simulink model "ofdm_txrx_alamouti"  (Simulink 6.3)
settings: Solver=ode45  StartTime=0.0  StopTime=45000  RelTol=1e-3
InitFcn:
  | ofdm_rx_alamouti_init
StartFcn:
  | ofdm_rx_alamouti_init
values from neighbouring files (symbols this model uses):
  CPLength = 16    [ofdm_tx_alamouti_init.m]
  CRCPolynomial = hex2dec('04c11db7')    [calcTxCRC.m]    (also in ofdm_tx_alamouti_init.m)
  hi = zeros(1,length(cci))    [ofdm_rx_alamouti_init.m]
  hr = zeros(1,length(ccr))    [ofdm_rx_alamouti_init.m]
  max_CP_length = 16    [ofdm_tx_alamouti_init.m]
  max_num_baseRateSymbols = 31    [ofdm_tx_alamouti_init.m]
  max_num_subcarriers = 64    [ofdm_tx_alamouti_init.m]
  max_num_trainingSymbols = 15    [ofdm_tx_alamouti_init.m]
  max_OFDM_symbols = 2047    [ofdm_tx_alamouti_init.m]
  modConstellation_qam16 = 0.75*[1 3 -1 -3]./3    [ofdm_tx_alamouti_init.m]
  modConstellation_qam256 = 0.9375*[3 1 5 7 11 9 13 15 -3 -1 -5 -7 -11 -9 -13 -15]./15    [ofdm_tx_alamouti_init.m]
  modConstellation_qam64 = 0.875*[3 1 5 7 -3 -1 -5 -7]./7    [ofdm_tx_alamouti_init.m]
  modConstellation_qpsk = [1 -1]./sqrt(2)    [ofdm_tx_alamouti_init.m]
  numBaseRateSymbols = 2    [ofdm_tx_alamouti_init.m]
  numFullRateSymbols = numFullRateSymbols + mod(numFullRateSymbols, 2)    [ofdm_tx_alamouti_init.m]
  numSubcarriers = 64    [ofdm_tx_alamouti_init.m]
  numTrainingSymbols = 4    [ofdm_tx_alamouti_init.m]
  pkt_crossCorr_thresh = 0.7    [ofdm_rx_alamouti_init.m]
  pkt_energy_thresh = 0    [ofdm_rx_alamouti_init.m]
  pkt_numPayloadBytes = 24+4+20+20+500    (= 568)    [ofdm_tx_alamouti_init.m]
  pktTiming_count_payloadStart = pktTiming_count_lsStart + pktTiming_ls_num*pktTiming_ls_length    [ofdm_rx_init_packetTimingControl.m]
  preamble = 6*[0 shortsyms_10 longsyms_2]    [ofdm_tx_alamouti_init.m]
  preamble_I = real(preamble)    [ofdm_tx_alamouti_init.m]
  preamble_Q = imag(preamble)    [ofdm_tx_alamouti_init.m]
  RAM_init_size = 4096    [ofdm_tx_alamouti_init.m]
  rx_longCorrCountLoad = 251-16    (= 235)    [ofdm_rx_alamouti_init.m]
  rx_longCorrThresh = 3000    [ofdm_rx_alamouti_init.m]
  RxDataScrambling_Seq = [40 198 78 63 82 173 102 245 48 111 172 115 147 230 216 93 72 65 62 2 205 242 122 90 128 83 105 97 73 10 5 252]    [ofdm_tx_alamouti_init.m]
  simOnly_numSamples = length(preamble)+( (numSubcarriers+CPLength)*(numTrainingSymbols + numBaseRateSymbols + numFullRateSymbols) )    [ofdm_tx_alamouti_init.m]
  train = [train train]    [ofdm_tx_alamouti_init.m]
  TxDataScrambling_Seq = [40 198 78 63 82 173 102 245 48 111 172 115 147 230 216 93 72 65 62 2 205 242 122 90 128 83 105 97 73 10 5 252]    [ofdm_tx_alamouti_init.m]
undefined symbols (not defined in the file or in its folder): CRC_table_gen, gcbh, interpFilter_4, xlBlockLoadCallback
libraries: xbsIndex_r4 x4373, dspmlti4 x4, dspsigops x4, commchan3 x2, simulink x2, commrflib2 x2, commsink2 x2, dspsrcs4 x1
(part 6 of 39)

== system ofdm_txrx_alamouti/OFDM Rx Alamouti/Equalizer & Packetizer/Packet_Constructor ==
  note: The index keeps track of the first unused location in the buffer, indexed from zero.
      |   I.e. if there are 5 bits in the buffer occupying 0 to 4, the index will be 5.
      | This is used to select which byte out of the 15 bits in the buffer will make it into the RAM block.
  I : Inport
  Q : Inport  Port=2
  Xk_index : Inport  Port=3
  Vin : Inport  Port=4
  Sym Index : Inport  Port=5
  Start : Inport  Port=6
  "  " : Subsystem  mask{period=1}
  >=8? : xbsIndex_r4/Slice  Orientation=up  nbits=1  boolean_output=off  mode=Lower Bit Location + Width  bit1=0  base1=MSB of Input  bit0=3  base0=LSB of Input  dbl_ovrd=off  block_type=slice
      settings: infoedit="Extracts a given range of bits from each input sample and presents it at the output.  The output type is ordinarily unsigned with binary point at zero, but can be Boolean when the slice is one bit wide.<P><P>Hardware notes: In hardware this block costs nothing."  block_version=VER_STRING_GOES_HERE
  AddSub1 : xbsIndex_r4/AddSub  mode=Subtraction  use_carryin=off  use_carryout=off  en=off  latency=0  precision=User Defined  arith_type=Unsigned  n_bits=4  bin_pt=0  quantization=Truncate  overflow=Wrap  dbl_ovrd=off  use_behavioral_HDL=on  pipelined=off  use_rpm=on  block_type=addsub
      settings: xl_area=[2 0 0 4 0 0 0]  block_version=VER_STRING_GOES_HERE
  AddSub3 : xbsIndex_r4/AddSub  mode=Addition  use_carryin=off  use_carryout=off  en=off  latency=0  precision=User Defined  arith_type=Unsigned  n_bits=4  bin_pt=0  quantization=Truncate  overflow=Wrap  dbl_ovrd=off  use_behavioral_HDL=on  pipelined=off  use_rpm=on  block_type=addsub
      settings: xl_area=[2 0 0 4 0 0 0]  block_version=VER_STRING_GOES_HERE
  Assert1 : xbsIndex_r4/Assert  Orientation=left  assert_type=off  type_source=Explicitly  arith_type="Signed  (2's comp)"  n_bits=16  bin_pt=14  assert_rate=on  rate_source=Explicitly  period=2  output_port=on  block_type=assert
      settings: infoedit=Asserts a user-defined sample rate and/or type on a signal.<P><P>Hardware notes: In hardware this block costs nothing.  block_version=VER_STRING_GOES_HERE
  Assert2 : xbsIndex_r4/Assert  assert_type=off  type_source=Explicitly  arith_type="Signed  (2's comp)"  n_bits=16  bin_pt=14  assert_rate=on  rate_source=Explicitly  period=2  output_port=on  block_type=assert
      settings: infoedit=Asserts a user-defined sample rate and/or type on a signal.<P><P>Hardware notes: In hardware this block costs nothing.  block_version=VER_STRING_GOES_HERE
  Barrel Shifter : Subsystem
  Convert2 : xbsIndex_r4/Convert  Orientation=down  arith_type=Boolean  n_bits=8  bin_pt=6  quantization=Truncate  overflow=Wrap  latency=0  dbl_ovrd=off  pipeline=off  block_type=convert
      settings: xl_area=[0 0 0 0 0 0 0]  block_version=VER_STRING_GOES_HERE
  Convert3 : xbsIndex_r4/Convert  Orientation=down  arith_type=Unsigned  n_bits=3  bin_pt=0  quantization=Truncate  overflow=Wrap  latency=0  dbl_ovrd=off  pipeline=off  block_type=convert
      settings: xl_area=[0 0 0 0 0 0 0]  block_version=VER_STRING_GOES_HERE
  Counter : xbsIndex_r4/Counter  cnt_type=Free Running  cnt_to=Inf  operation=Up  start_count=0  cnt_by_val=1  arith_type=Unsigned  n_bits=ceil(log2(RAM_init_size))  bin_pt=0  load_pin=off  rst=on  en=on  explicit_period=on  period=2  dbl_ovrd=off  use_behavioral_HDL=off  use_rpm=on  block_type=counter
      settings: xl_area=[5 10 0 10 0 0 0]  block_version=VER_STRING_GOES_HERE
  Data Buffer : Subsystem
  Delay1 : xbsIndex_r4/Delay  en=off  latency=1  dbl_ovrd=off  reg_retiming=off  block_type=delay
      settings: infoedit=Hardware notes: A delay line is a chain, each link of which is an SRL16 followed by a flip-flop. If register retiming is enabled, the delay line is a chain of flip-flops.  xl_area=[1 1 0 0 0 0 0]  block_version=VER_STRING_GOES_HERE
  Delay2 : xbsIndex_r4/Delay  en=off  latency=1  dbl_ovrd=off  reg_retiming=off  block_type=delay
      settings: infoedit=Hardware notes: A delay line is a chain, each link of which is an SRL16 followed by a flip-flop. If register retiming is enabled, the delay line is a chain of flip-flops.  xl_area=[1 1 0 0 0 0 0]  block_version=VER_STRING_GOES_HERE
  Delay3 : xbsIndex_r4/Delay  en=off  latency=1  dbl_ovrd=off  reg_retiming=off  block_type=delay
      settings: infoedit=Hardware notes: A delay line is a chain, each link of which is an SRL16 followed by a flip-flop. If register retiming is enabled, the delay line is a chain of flip-flops.  xl_area=[1 1 0 0 0 0 0]  block_version=VER_STRING_GOES_HERE
  Detection Disable : Subsystem  Orientation=left
  Hard Decision : Subsystem
  Inverter : xbsIndex_r4/Inverter  en=off  latency=0  dbl_ovrd=off  block_type=inv
      settings: xl_area=[0 0 0 0 0 0 0]  block_version=VER_STRING_GOES_HERE
  Inverter1 : xbsIndex_r4/Inverter  en=off  latency=0  dbl_ovrd=off  block_type=inv
      settings: xl_area=[0 0 0 0 0 0 0]  block_version=VER_STRING_GOES_HERE
  Logical1 : xbsIndex_r4/Logical  Orientation=down  logical_function=AND  inputs=3  en=off  latency=0  precision=Full  arith_type=Unsigned  n_bits=8  bin_pt=2  align_bp=on  dbl_ovrd=off  block_type=logical
      settings: xl_area=[1 0 0 1 0 0 0]  block_version=VER_STRING_GOES_HERE
  Logical2 : xbsIndex_r4/Logical  logical_function=AND  inputs=3  en=off  latency=0  precision=Full  arith_type=Unsigned  n_bits=8  bin_pt=2  align_bp=on  dbl_ovrd=off  block_type=logical
      settings: xl_area=[1 0 0 1 0 0 0]  block_version=VER_STRING_GOES_HERE
  Logical3 : xbsIndex_r4/Logical  logical_function=AND  inputs=2  en=off  latency=0  precision=Full  arith_type=Unsigned  n_bits=8  bin_pt=2  align_bp=on  dbl_ovrd=off  block_type=logical
      settings: xl_area=[1 0 0 1 0 0 0]  block_version=VER_STRING_GOES_HERE
  Logical4 : xbsIndex_r4/Logical  logical_function=OR  inputs=3  en=off  latency=0  precision=Full  arith_type=Unsigned  n_bits=8  bin_pt=2  align_bp=on  dbl_ovrd=off  block_type=logical
      settings: xl_area=[4 0 0 4 0 0 0]  block_version=VER_STRING_GOES_HERE
  Modulation RAM : Subsystem
  Pkt Constructor Guts : Scope  NumInputPorts=6
      settings: TimeRange=9000  YMin=0~0~0~0~0~0  YMax=3~2~40000~6~300~1  SaveName=ScopeData34
  Register : xbsIndex_r4/Register  init=0  rst=off  en=off  dbl_ovrd=off  block_type=register
      settings: block_version=8.2.02
  Relational : xbsIndex_r4/Relational  mode=a<b  en=off  latency=0  dbl_ovrd=off  block_type=relational
      settings: xl_area=[6 0 0 11 0 0 0]  block_version=VER_STRING_GOES_HERE
  Shift8 : xbsIndex_r4/Shift  Orientation=left  shift_dir=Left  shift_bits=3  en=off  latency=0  precision=User Defined  arith_type=Unsigned  n_bits=4  bin_pt=0  quantization=Truncate  overflow=Wrap  dbl_ovrd=off  block_type=shift
      settings: xl_area=[0 0 0 0 0 0 0]  block_version=VER_STRING_GOES_HERE
  Slicer : Subsystem
  done{1..4,6..7} (6 alike) : xbsIndex_r4/Gateway Out  hdl_port=off  timing_constraint=None  locs_specified=off  LOCs={}  block_type=gatewayout
      settings: infoedit="Gateway out block.  Converts Xilinx fixed point inputs into ouputs of type Simulink integer, double, or fixed point.<P><P>Hardware notes:  In hardware these blocks become top level output ports or are discarded, depending on how they are configured."  xl_area=[0 0 0 0 1 0 0]  block_version=VER_STRING_GOES_HERE
  index acc1 : xbsIndex_r4/Accumulator  operation=Add  n_bits=4  overflow=Wrap  scale=1  rst=on  hasbypass=off  en=on  dbl_ovrd=off  use_behavioral_HDL=on  block_type=accum
      settings: infoedit="Adder or subtractor-based accumulator.   Output type and binary point position match the input.<P><P>Hardware notes: When \"Reinitialize with input 'b' on reset\" is selected, the accumulator is forced to run at the system rate even if the input 'b' is running at a slower rate."  xl_area=[2 4 0 4 0 0 0]  block_version=VER_STRING_GOES_HERE
  done : Outport
  wires:
  Delay2/1 -> AddSub1/1, AddSub3/1, Barrel Shifter/1(ShiftSel), done7/1
  Data Buffer/1(numBytes) -> Relational/2
  Logical4/1 -> index acc1/2, Data Buffer/4(Rst), Counter/1
  AddSub1/1 -> index acc1/1
  Shift8/1 -> AddSub1/2
  index acc1/1 -> AddSub3/2
  AddSub3/1 -> Assert2/1
  >=8?/1 -> Shift8/1, Convert2/1
  Barrel Shifter/1(Q) -> Slicer/2(D), done1/1
  Logical2/1 -> Data Buffer/2(Payload_WE)
  Slicer/1(Q) -> done3/1, Data Buffer/3(Payload_Data)
  "  "/1(pulse) -> Register/1
  Inverter/1 -> "  "/1(signal)
  Convert2/1 -> Logical2/2, Logical1/2
  Logical1/1 -> Counter/2
  Logical3/1 -> Logical2/1, Logical1/3, Inverter/1
  Counter/1 -> Data Buffer/1(Payload_Addr), Relational/1
  Convert3/1 -> Slicer/1(SliceSel), done2/1
  Modulation RAM/1(mod) -> Hard Decision/1(Mod Sel), Delay2/1
  Xk_index/1 -> Modulation RAM/1(Xk_index)
  Sym Index/1 -> Modulation RAM/2(Sym Index)
  Hard Decision/1(bits) -> Barrel Shifter/2(D), done6/1
  I/1 -> Hard Decision/2(I)
  Q/1 -> Hard Decision/3(Q)
  done6/1 -> Pkt Constructor Guts/1
  done7/1 -> Pkt Constructor Guts/2
  done1/1 -> Pkt Constructor Guts/3
  done2/1 -> Pkt Constructor Guts/4
  done3/1 -> Pkt Constructor Guts/5
  Start/1 -> Logical4/1
  [rx_global_reset] -> Logical4/2, Logical4/3
  Data Buffer/3(CRCError) -> Detection Disable/1(CRC-Err)
  Assert2/1 -> Convert3/1, >=8?/1
  Vin/1 -> Delay3/1
  Delay1/1 -> Barrel Shifter/3(en), index acc1/3, Logical1/1, Logical2/3, done4/1
  done4/1 -> Pkt Constructor Guts/6
  Delay3/1 -> Delay1/1
  Register/1 -> done/1, Detection Disable/2(PktDone)
  Data Buffer/2(modMask) -> Modulation RAM/3(FullRateMask)
  Data Buffer/4(HdrError) -> Detection Disable/3(HdrError), Assert1/1
  Relational/1 -> Logical3/1
  Inverter1/1 -> Logical3/2
  Assert1/1 -> Inverter1/1

== system ofdm_txrx_alamouti/OFDM Rx Alamouti/Equalizer & Packetizer/Phase Adjust ==
  Phase : Inport
  Y_I : Inport  Port=2
  Y_Q : Inport  Port=3
  H_I : Inport  Port=4
  H_Q : Inport  Port=5
  Vin : Inport  Port=6
  Sym Index : Inport  Port=7
  SubCar Index : Inport  Port=8
  Bus Creator : Subsystem
  Bus Creator1 : Subsystem
  Bus Selector : Subsystem
  Bus Selector2 : Subsystem
  ComplexMult_x1 : Subsystem
  ComplexMult_x2 : Subsystem
  Delay{1..4,8..11} (8 alike) : xbsIndex_r4/Delay  en=off  latency=1  dbl_ovrd=off  reg_retiming=off  block_type=delay
      settings: infoedit=Hardware notes: A delay line is a chain, each link of which is an SRL16 followed by a flip-flop. If register retiming is enabled, the delay line is a chain of flip-flops.  xl_area=[0, 0, 0, 0, 0, 0, 0]  block_version=VER_STRING_GOES_HERE
  Delay{5..7,12..15} (7 alike) : xbsIndex_r4/Delay  en=off  latency=4  dbl_ovrd=off  reg_retiming=off  block_type=delay
      settings: infoedit=Hardware notes: A delay line is a chain, each link of which is an SRL16 followed by a flip-flop. If register retiming is enabled, the delay line is a chain of flip-flops.  xl_area=[0, 0, 0, 0, 0, 0, 0]  block_version=VER_STRING_GOES_HERE
  Phase Adjust : Scope  NumInputPorts=7
      settings: TimeRange=1800  YMin=0~-0.005~0.949971~-0.4~-0.5~-0.4~-0.5  YMax=0.003~0~1.04997~0.3~0.3~0.3~0.3  SaveName=ScopeData67
  Phase Calc : Subsystem
  Pre-PhaseAdj : commsink2/Discrete-Time Scatter Plot Scope  Tag=ScatterPlot  sampPerSymb=1  offsetEye=0  numTraces=1500  numNewFrames=10  LineMarkers=.  LineColors=b  fading=on  render=on  AxisGrid=on  xMin=-1.10677642822266  xMax=1.11238838195801  yMin=-1.10849597930908  yMax=1.11066883087158  inphaseLabel=In-phase Amplitude  quadratureLabel=Quadrature Amplitude  openScopeAtSimStart=off  FrameNumber=off  FigPos=[360 362 560 560]  figTitle=Scatter Plot  numLinesMax=8  block_type_=scatter
  Real-Imag to Complex : RealImagToComplex
  SineCosine : xbsIndex_r4/SineCosine  mode=Sine and Cosine  neg_sin=on  neg_cos=off  n_bits=16  sym_output=off  en=off  latency=1  dbl_ovrd=off  explicit_period=off  period=1  mem_type=Block RAM  pipeline=off  use_rpm=on  block_type=sincos
      settings: block_version=8.2.02
  done{1..2,6..10} (7 alike) : xbsIndex_r4/Gateway Out  hdl_port=off  timing_constraint=None  locs_specified=off  LOCs={}  block_type=gatewayout
      settings: infoedit="Gateway out block.  Converts Xilinx fixed point inputs into ouputs of type Simulink integer, double, or fixed point.<P><P>Hardware notes:  In hardware these blocks become top level output ports or are discarded, depending on how they are configured."  xl_area=[0 0 0 0 1 0 0]  block_version=VER_STRING_GOES_HERE
  " Y_I" : Outport
  " Y_Q" : Outport  Port=2
  " H-I" : Outport  Port=3
  " H-Q" : Outport  Port=4
  " SubCar Index" : Outport  Port=5
  Vout : Outport  Port=6
  " Sym Index" : Outport  Port=7
  wires:
  SineCosine/2 -> ComplexMult_x1/3(Re[B]), ComplexMult_x2/1(Re[A]), done1/1
  Vin/1 -> Delay5/1
  Sym Index/1 -> Delay6/1
  SubCar Index/1 -> Delay7/1
  Delay7/1 -> " SubCar Index"/1
  Delay6/1 -> " Sym Index"/1
  Delay5/1 -> Vout/1
  Delay1/1 -> ComplexMult_x1/1(Re[A])
  Delay2/1 -> ComplexMult_x1/2(Im[A])
  SineCosine/1 -> ComplexMult_x1/4(Im[B]), ComplexMult_x2/2(Im[A]), done7/1
  done6/1 -> Phase Adjust/1
  done7/1 -> Phase Adjust/2
  done1/1 -> Phase Adjust/3
  done8/1 -> Phase Adjust/4, Real-Imag to Complex/1
  done9/1 -> Phase Adjust/5, Real-Imag to Complex/2
  done10/1 -> Phase Adjust/6
  done2/1 -> Phase Adjust/7
  Real-Imag to Complex/1 -> Pre-PhaseAdj/1
  Phase Calc/1(" Phase") -> SineCosine/1, done6/1
  Phase/1 -> Phase Calc/1(Phase)
  Delay3/1 -> Delay2/1
  Delay4/1 -> Delay1/1
  H_I/1 -> Bus Selector/1(H-I)
  H_Q/1 -> Bus Selector/2(H-Q)
  Y_I/1 -> Bus Selector2/1(Y_I)
  Y_Q/1 -> Bus Selector2/2(Y_Q)
  Bus Creator/1(I) -> " H-I"/1
  Bus Creator/2(Q) -> " H-Q"/1
  Bus Creator1/1(" I") -> " Y_I"/1
  Bus Creator1/2(" Q") -> " Y_Q"/1
  Bus Selector/1(AA_I) -> Delay12/1
  Bus Selector/2(AA_Q) -> Delay13/1
  Bus Selector/3(BA_I) -> Delay14/1
  Bus Selector/4(BA_Q) -> Delay15/1
  Delay12/1 -> Bus Creator/1(AA-I)
  Delay13/1 -> Bus Creator/2(AA-Q)
  Delay14/1 -> Bus Creator/3(BA-I)
  Delay15/1 -> Bus Creator/4(BA-Q)
  Bus Selector2/1(R0_I) -> Delay4/1
  Bus Selector2/2(R0_Q) -> Delay3/1
  Delay10/1 -> Delay9/1
  Delay11/1 -> Delay8/1
  Bus Selector2/3(R1_I) -> Delay11/1
  Bus Selector2/4(R1_Q) -> Delay10/1
  Delay8/1 -> ComplexMult_x2/3(Re[B]), done8/1
  Delay9/1 -> ComplexMult_x2/4(Im[B]), done9/1
  ComplexMult_x1/1(Re) -> Bus Creator1/1(R0_I)
  ComplexMult_x1/2(Im) -> Bus Creator1/2(R0_Q)
  ComplexMult_x2/1(Re) -> Bus Creator1/3(R1_I), done10/1
  ComplexMult_x2/2(Im) -> Bus Creator1/4(R1_Q), done2/1

== system ofdm_txrx_alamouti/OFDM Rx Alamouti/Equalizer & Packetizer/Phase Noise Tracker ==
  Phase Err : Inport
  Reset : Inport  Port=2
  Vin : Inport  Port=3
  Sym Index : Inport  Port=4
  Coarse Tracking : Subsystem
  Fine Tracking : Subsystem
  theta : Outport
  wires:
  Phase Err/1 -> Coarse Tracking/1(Phase Err)
  Reset/1 -> Coarse Tracking/2(Rst), Fine Tracking/4(Rst)
  Vin/1 -> Coarse Tracking/3(Vin)
  Coarse Tracking/1(phi) -> Fine Tracking/1(phi)
  Coarse Tracking/2(theta_coarse) -> Fine Tracking/2(theta_coarse)
  Sym Index/1 -> Fine Tracking/5(Sym Index)
  Coarse Tracking/3(vout) -> Fine Tracking/3(Vin)
  Fine Tracking/1(theta) -> theta/1

== system ofdm_txrx_alamouti/OFDM Rx Alamouti/Equalizer & Packetizer/Pilot_Extractor ==
  Y_I : Inport
  Y_Q : Inport  Port=2
  H-I : Inport  Port=3
  H-Q : Inport  Port=4
  Sym Index : Inport  Port=5
  Xk_index : Inport  Port=6
  Vin : Inport  Port=7
  rst : Inport  Port=8
  1LSB1 : xbsIndex_r4/Slice  nbits=1  boolean_output=on  mode=Lower Bit Location + Width  bit1=0  base1=MSB of Input  bit0=0  base0=LSB of Input  dbl_ovrd=off  block_type=slice
      settings: infoedit="Extracts a given range of bits from each input sample and presents it at the output.  The output type is ordinarily unsigned with binary point at zero, but can be Boolean when the slice is one bit wide.<P><P>Hardware notes: In hardware this block costs nothing."  block_version=VER_STRING_GOES_HERE
  Complex to Magnitude-Angle : ComplexToMagnitudeAngle
  Delay1 : xbsIndex_r4/Delay  en=off  latency=1  dbl_ovrd=off  reg_retiming=off  block_type=delay
      settings: infoedit=Hardware notes: A delay line is a chain, each link of which is an SRL16 followed by a flip-flop. If register retiming is enabled, the delay line is a chain of flip-flops.  block_version=VER_STRING_GOES_HERE
  Delay2 : xbsIndex_r4/Delay  en=off  latency=2  dbl_ovrd=off  reg_retiming=off  block_type=delay
      settings: infoedit=Hardware notes: A delay line is a chain, each link of which is an SRL16 followed by a flip-flop. If register retiming is enabled, the delay line is a chain of flip-flops.  xl_area=[0, 0, 0, 0, 0, 0, 0]  block_version=VER_STRING_GOES_HERE
  Delay4 : xbsIndex_r4/Delay  en=off  latency=1  dbl_ovrd=off  reg_retiming=off  block_type=delay
      settings: infoedit=Hardware notes: A delay line is a chain, each link of which is an SRL16 followed by a flip-flop. If register retiming is enabled, the delay line is a chain of flip-flops.  xl_area=[0, 0, 0, 0, 0, 0, 0]  block_version=VER_STRING_GOES_HERE
  Delay5 : xbsIndex_r4/Delay  en=off  latency=2  dbl_ovrd=off  reg_retiming=off  block_type=delay
      settings: infoedit=Hardware notes: A delay line is a chain, each link of which is an SRL16 followed by a flip-flop. If register retiming is enabled, the delay line is a chain of flip-flops.  xl_area=[0, 0, 0, 0, 0, 0, 0]  block_version=VER_STRING_GOES_HERE
  Gateway Out{1..5} (5 alike) : xbsIndex_r4/Gateway Out  hdl_port=off  timing_constraint=None  locs_specified=off  LOCs={}  block_type=gatewayout
      settings: infoedit="Gateway out block.  Converts Xilinx fixed point inputs into ouputs of type Simulink integer, double, or fixed point.<P><P>Hardware notes:  In hardware these blocks become top level output ports or are discarded, depending on how they are configured."  block_version=VER_STRING_GOES_HERE
  Logical2 : xbsIndex_r4/Logical  logical_function=AND  inputs=2  en=off  latency=0  precision=Full  arith_type=Unsigned  n_bits=8  bin_pt=2  align_bp=on  dbl_ovrd=off  block_type=logical
      settings: xl_area=[2 0 0 2 0 0 0]  block_version=VER_STRING_GOES_HERE
  Phase Calc & Combining : Subsystem
  Pilot Descrambling : Subsystem
  Pilot Extractor : Scope  NumInputPorts=6
      settings: TimeRange=8500  YMin=-1~-1~-1~0~-1~-1  YMax=1~1~1~1~1~1  SaveName=ScopeData25
  Pilot Phase Equalizer : Subsystem
  Real-Imag to Complex : RealImagToComplex
  Register : xbsIndex_r4/Register  init=0  rst=off  en=on  dbl_ovrd=off  block_type=register
      settings: block_version=VER_STRING_GOES_HERE
  Register1 : xbsIndex_r4/Register  init=0  rst=off  en=on  dbl_ovrd=off  block_type=register
      settings: block_version=VER_STRING_GOES_HERE
  Register10 : xbsIndex_r4/Register  init=0  rst=on  en=on  dbl_ovrd=off  block_type=register
      settings: block_version=VER_STRING_GOES_HERE
  Register12 : xbsIndex_r4/Register  init=0  rst=off  en=on  dbl_ovrd=off  block_type=register
      settings: block_version=VER_STRING_GOES_HERE
  Relational1 : xbsIndex_r4/Relational  mode=a<b  en=off  latency=0  dbl_ovrd=off  block_type=relational
      settings: xl_area=[6 0 0 11 0 0 0]  block_version=VER_STRING_GOES_HERE
  S-R Latch : Subsystem
  Shift : xbsIndex_r4/Shift  shift_dir=Left  shift_bits=1  en=off  latency=0  precision=User Defined  arith_type=Unsigned  n_bits=1+ceil(log2(max_num_trainingSymbols))  bin_pt=0  quantization=Truncate  overflow=Flag as error  dbl_ovrd=off  block_type=shift
      settings: block_version=VER_STRING_GOES_HERE
  posedge : Subsystem
  Phase Err : Outport
  Rst : Outport  Port=2
  Vout : Outport  Port=3
  wires:
  Pilot Phase Equalizer/2(Q) -> Pilot Descrambling/4("Q ")
  rst/1 -> Pilot Descrambling/2(Rst), S-R Latch/1(S), Phase Calc & Combining/1(Rst)
  Register/1 -> Phase Calc & Combining/2(I), Gateway Out5/1
  Register1/1 -> Gateway Out4/1, Phase Calc & Combining/3(Q)
  Delay2/1 -> Pilot Descrambling/1(Sym Index), Relational1/2
  Phase Calc & Combining/1(PhaseEst) -> Register10/1
  [regRx_numTrainingSymbols] -> Relational1/1, Shift/1
  Gateway Out5/1 -> Pilot Extractor/1, Real-Imag to Complex/1
  Gateway Out4/1 -> Pilot Extractor/2, Real-Imag to Complex/2
  Gateway Out3/1 -> Pilot Extractor/3
  Gateway Out2/1 -> Pilot Extractor/4
  Gateway Out1/1 -> Pilot Extractor/5
  Relational1/1 -> posedge/1(D), Logical2/1, Gateway Out2/1
  S-R Latch/1(D) -> Register10/2, Rst/1
  posedge/1(Q) -> S-R Latch/2(R)
  Logical2/1 -> Register10/3, Delay1/1, Gateway Out3/1
  Delay1/1 -> Vout/1
  Register10/1 -> Phase Err/1, Gateway Out1/1
  Real-Imag to Complex/1 -> Complex to Magnitude-Angle/1
  Complex to Magnitude-Angle/2 -> Pilot Extractor/6
  Y_Q/1 -> Pilot Phase Equalizer/3(Y_Q)
  Y_I/1 -> Pilot Phase Equalizer/2(Y_I)
  H-Q/1 -> Pilot Phase Equalizer/5(H_Q)
  H-I/1 -> Pilot Phase Equalizer/4(H_I)
  Sym Index/1 -> 1LSB1/1, Delay2/1
  1LSB1/1 -> Pilot Phase Equalizer/1(SlotSel)
  Xk_index/1 -> Delay5/1
  Pilot Phase Equalizer/1(I) -> Pilot Descrambling/3("I ")
  Pilot Descrambling/3(PilotEn) -> Register1/2, Register/2, Register12/2, Delay4/1
  Pilot Phase Equalizer/3(mag(H )) -> Register12/1
  Register12/1 -> Phase Calc & Combining/4(mag(H))
  Delay4/1 -> Phase Calc & Combining/5(Vin)
  Phase Calc & Combining/2(Vout) -> Logical2/2
  Pilot Descrambling/1(I) -> Register/1
  Pilot Descrambling/2(Q) -> Register1/1
  Delay5/1 -> Pilot Descrambling/5(FFT Index)
  Vin/1 -> Pilot Descrambling/6(Vin)

== system ofdm_txrx_alamouti/OFDM Rx Alamouti/Equalizer & Packetizer/Scaling ==
  note: This block scales the equalized constellation so it can be easily sliced/demapped for hard decisions,
  Eq-I : Inport
  Eq-Q : Inport  Port=2
  Xk_index : Inport  Port=3
  Vin : Inport  Port=4
  Sym Index : Inport  Port=5
  " To_UFix16_11" : xbsIndex_r4/Reinterpret  force_arith_type=on  arith_type=Unsigned  force_bin_pt=on  bin_pt=11  block_type=cast
      settings: infoedit="Changes type of samples without altering their binary representation.<P><P>Hardware notes: In hardware this block costs nothing.<P><P>Example:  Suppose the input is 6 bits wide, signed, with 2 fractional bits, and the output is forced to unsigned with 0 fractional bits.  Then an input of -2.0 (1110.00 in binary 2's complement) becomes an output of 56 (111000 in binary)."  block_version=VER_STRING_GOES_HERE
  16LSB : xbsIndex_r4/Slice  nbits=16  boolean_output=off  mode=Lower Bit Location + Width  bit1=0  base1=LSB of Input  bit0=0  base0=LSB of Input  dbl_ovrd=off  block_type=slice
      settings: infoedit="Extracts a given range of bits from each input sample and presents it at the output.  The output type is ordinarily unsigned with binary point at zero, but can be Boolean when the slice is one bit wide.<P><P>Hardware notes: In hardware this block costs nothing."  block_version=VER_STRING_GOES_HERE
  16MSB : xbsIndex_r4/Slice  nbits=16  boolean_output=off  mode=Lower Bit Location + Width  bit1=0  base1=LSB of Input  bit0=16  base0=LSB of Input  dbl_ovrd=off  block_type=slice
      settings: infoedit="Extracts a given range of bits from each input sample and presents it at the output.  The output type is ordinarily unsigned with binary point at zero, but can be Boolean when the slice is one bit wide.<P><P>Hardware notes: In hardware this block costs nothing."  block_version=VER_STRING_GOES_HERE
  1LSB : xbsIndex_r4/Slice  nbits=1  boolean_output=off  mode=Lower Bit Location + Width  bit1=0  base1=MSB of Input  bit0=0  base0=LSB of Input  dbl_ovrd=off  block_type=slice
      settings: infoedit="Extracts a given range of bits from each input sample and presents it at the output.  The output type is ordinarily unsigned with binary point at zero, but can be Boolean when the slice is one bit wide.<P><P>Hardware notes: In hardware this block costs nothing."  block_version=VER_STRING_GOES_HERE
  Delay1 : xbsIndex_r4/Delay  en=off  latency=1  dbl_ovrd=off  reg_retiming=off  block_type=delay
      settings: infoedit=Hardware notes: A delay line is a chain, each link of which is an SRL16 followed by a flip-flop. If register retiming is enabled, the delay line is a chain of flip-flops.  xl_area=[0, 0, 0, 0, 0, 0, 0]  block_version=VER_STRING_GOES_HERE
  Delay3 : xbsIndex_r4/Delay  en=off  latency=1  dbl_ovrd=off  reg_retiming=off  block_type=delay
      settings: infoedit=Hardware notes: A delay line is a chain, each link of which is an SRL16 followed by a flip-flop. If register retiming is enabled, the delay line is a chain of flip-flops.  xl_area=[0, 0, 0, 0, 0, 0, 0]  block_version=VER_STRING_GOES_HERE
  Delay4 : xbsIndex_r4/Delay  en=off  latency=1  dbl_ovrd=off  reg_retiming=off  block_type=delay
      settings: infoedit=Hardware notes: A delay line is a chain, each link of which is an SRL16 followed by a flip-flop. If register retiming is enabled, the delay line is a chain of flip-flops.  xl_area=[0, 0, 0, 0, 0, 0, 0]  block_version=VER_STRING_GOES_HERE
  Down Sample1 : xbsIndex_r4/Down Sample  sample_ratio=2  sample_phase="Last Value of Frame  (most efficient)"  en=off  latency=1  dbl_ovrd=off  block_type=dsamp
      settings: block_version=VER_STRING_GOES_HERE
  Mult : xbsIndex_r4/Mult  precision=User Defined  arith_type="Signed  (2's comp)"  n_bits=symbol_unmap_nb  bin_pt=symbol_unmap_bp  quantization="Round  (unbiased: +/- Inf)"  overflow=Saturate  en=off  latency=1  dbl_ovrd=off  use_behavioral_HDL=off  use_embedded=on  opt=Speed  optimum_pipeline=off  use_rpm=off  placement_style=Rectangular shape  pipeline=on  block_type=mult
      settings: infoedit=Hardware notes: To use the internal pipeline stage of the dedicated multiplier you must select 'Pipeline for maximum performance'.  xl_area=[12 0 0 20 0 1 0]  block_version=VER_STRING_GOES_HERE
  Mult1 : xbsIndex_r4/Mult  precision=User Defined  arith_type="Signed  (2's comp)"  n_bits=symbol_unmap_nb  bin_pt=symbol_unmap_bp  quantization="Round  (unbiased: +/- Inf)"  overflow=Saturate  en=off  latency=1  dbl_ovrd=off  use_behavioral_HDL=off  use_embedded=on  opt=Speed  optimum_pipeline=off  use_rpm=off  placement_style=Rectangular shape  pipeline=on  block_type=mult
      settings: infoedit=Hardware notes: To use the internal pipeline stage of the dedicated multiplier you must select 'Pipeline for maximum performance'.  xl_area=[12 0 0 20 0 1 0]  block_version=VER_STRING_GOES_HERE
  Mux : xbsIndex_r4/Mux  inputs=2  en=off  latency=0  precision=Full  arith_type=Unsigned  n_bits=16  bin_pt=14  quantization=Truncate  overflow=Wrap  dbl_ovrd=off  block_type=mux
      settings: block_version=VER_STRING_GOES_HERE
  Rx_Constellation_Scaling : xbsIndex_r4/From Register  shared_memory_name='Rx_Constellation_Scaling'  init=rx_postEq_scaling  period=1  ownership=Locally owned and initialized  arith_type=Unsigned  n_bits=32  bin_pt=0  dbl_ovrd=off  block_type=fromreg
      settings: AttributesFormatString=<< %<shared_memory_name> >>  infoedit="Register block that reads data to a shared memory register.  Delay of one sample period."  block_version=VER_STRING_GOES_HERE
  Scaled Constellation : Scope  NumInputPorts=4
      settings: TimeRange=1800  YMin=96~171~0~-1  YMax=108~182~1~1  SaveName=ScopeData56
  To_UFix16_11 : xbsIndex_r4/Reinterpret  force_arith_type=on  arith_type=Unsigned  force_bin_pt=on  bin_pt=11  block_type=cast
      settings: infoedit="Changes type of samples without altering their binary representation.<P><P>Hardware notes: In hardware this block costs nothing.<P><P>Example:  Suppose the input is 6 bits wide, signed, with 2 fractional bits, and the output is forced to unsigned with 0 fractional bits.  Then an input of -2.0 (1110.00 in binary 2's complement) becomes an output of 56 (111000 in binary)."  block_version=VER_STRING_GOES_HERE
  done{2..5} (4 alike) : xbsIndex_r4/Gateway Out  hdl_port=off  timing_constraint=None  locs_specified=off  LOCs={}  block_type=gatewayout
      settings: infoedit="Gateway out block.  Converts Xilinx fixed point inputs into ouputs of type Simulink integer, double, or fixed point.<P><P>Hardware notes:  In hardware these blocks become top level output ports or are discarded, depending on how they are configured."  xl_area=[0 0 0 0 1 0 0]  block_version=VER_STRING_GOES_HERE
  I : Outport
  Q : Outport  Port=2
  " Xk_index" : Outport  Port=3
  Vout : Outport  Port=4
  " Sym Index" : Outport  Port=5
  wires:
  Eq-Q/1 -> Mult1/2
  Eq-I/1 -> Mult/2
  Mult1/1 -> done4/1, Q/1
  Mult/1 -> done3/1, I/1
  Mux/1 -> Mult1/1, Mult/1, done2/1
  1LSB/1 -> Mux/1, done5/1
  " To_UFix16_11"/1 -> Mux/3
  To_UFix16_11/1 -> Mux/2
  Xk_index/1 -> Delay1/1
  Delay1/1 -> " Xk_index"/1
  Vin/1 -> Delay3/1
  Delay3/1 -> Vout/1
  Sym Index/1 -> Delay4/1, 1LSB/1
  Delay4/1 -> " Sym Index"/1
  Rx_Constellation_Scaling/1 -> Down Sample1/1
  16LSB/1 -> To_UFix16_11/1
  16MSB/1 -> " To_UFix16_11"/1
  Down Sample1/1 -> 16LSB/1, 16MSB/1
  done3/1 -> Scaled Constellation/1
  done4/1 -> Scaled Constellation/2
  done2/1 -> Scaled Constellation/3
  done5/1 -> Scaled Constellation/4

== system ofdm_txrx_alamouti/OFDM Rx Alamouti/Equalizer & Packetizer/posedge ==
  note: Rising Edge Detection
  D : Inport
  Delay : xbsIndex_r4/Delay  en=off  latency=1  dbl_ovrd=off  reg_retiming=off  block_type=delay
      settings: infoedit=Hardware notes: A delay line is a chain, each link of which is an SRL16 followed by a flip-flop. If register retiming is enabled, the delay line is a chain of flip-flops.  xl_area=[1 1 0 0 0 0 0]  block_version=VER_STRING_GOES_HERE
  Inverter : xbsIndex_r4/Inverter  en=off  latency=0  dbl_ovrd=off  block_type=inv
      settings: xl_area=[0 0 0 0 0 0 0]  block_version=VER_STRING_GOES_HERE
  Logical1 : xbsIndex_r4/Logical  logical_function=AND  inputs=2  en=off  latency=0  precision=Full  arith_type=Unsigned  n_bits=8  bin_pt=2  align_bp=on  dbl_ovrd=off  block_type=logical
      settings: xl_area=[1 0 0 1 0 0 0]  block_version=VER_STRING_GOES_HERE
  Q : Outport
  wires:
  Delay/1 -> Inverter/1
  Inverter/1 -> Logical1/1
  Logical1/1 -> Q/1
  D/1 -> Delay/1, Logical1/2

== system ofdm_txrx_alamouti/OFDM Rx Alamouti/FFT & Chan Est/Addr Gen ==
  note: Delay the beginning of the read address
      | generation by one cycle, to allow the FFT
      | outputs to be written one cycle before
      | they are read from the buffers.
      | |
      | V
  FFT Done : Inport
  Payload : Inport  Port=2
  NotTraining : Inport  Port=3
  1MSB : xbsIndex_r4/Slice  nbits=1  boolean_output=on  mode=Upper Bit Location + Width  bit1=0  base1=MSB of Input  bit0=0  base0=MSB of Input  dbl_ovrd=off  block_type=slice
      settings: infoedit="Extracts a given range of bits from each input sample and presents it at the output.  The output type is ordinarily unsigned with binary point at zero, but can be Boolean when the slice is one bit wide.<P><P>Hardware notes: In hardware this block costs nothing."  block_version=VER_STRING_GOES_HERE
  6LSB : xbsIndex_r4/Slice  nbits=6  boolean_output=off  mode=Lower Bit Location + Width  bit1=0  base1=LSB of Input  bit0=0  base0=LSB of Input  dbl_ovrd=off  block_type=slice
      settings: infoedit="Extracts a given range of bits from each input sample and presents it at the output.  The output type is ordinarily unsigned with binary point at zero, but can be Boolean when the slice is one bit wide.<P><P>Hardware notes: In hardware this block costs nothing."  block_version=VER_STRING_GOES_HERE
  Constant1 : xbsIndex_r4/Constant  Orientation=left  arith_type=Unsigned  const=numSubcarriers*2-1  n_bits=ceil(log2(numSubcarriers*2))  bin_pt=0  explicit_period=off  period=1  equ=P=C  opselect=C  inp2=PCIN>>17  opr=+  inp1=P  carry=CIN  dbl_ovrd=off  block_type=constant
      settings: block_version=VER_STRING_GOES_HERE
  Counter : xbsIndex_r4/Counter  cnt_type=Free Running  cnt_to=127  operation=Up  start_count=0  cnt_by_val=1  arith_type=Unsigned  n_bits=ceil(log2(numSubcarriers*2))  bin_pt=0  load_pin=off  rst=on  en=on  explicit_period=off  period=2  dbl_ovrd=off  use_behavioral_HDL=off  use_rpm=on  block_type=counter
      settings: xl_area=[6 12 0 12 0 0 0]  block_version=VER_STRING_GOES_HERE
  Delay1 : xbsIndex_r4/Delay  en=off  latency=1  dbl_ovrd=off  reg_retiming=off  block_type=delay
      settings: infoedit=Hardware notes: A delay line is a chain, each link of which is an SRL16 followed by a flip-flop. If register retiming is enabled, the delay line is a chain of flip-flops.  block_version=VER_STRING_GOES_HERE
  Delay2 : xbsIndex_r4/Delay  en=off  latency=1  dbl_ovrd=off  reg_retiming=off  block_type=delay
      settings: infoedit=Hardware notes: A delay line is a chain, each link of which is an SRL16 followed by a flip-flop. If register retiming is enabled, the delay line is a chain of flip-flops.  block_version=VER_STRING_GOES_HERE
  FFT Output Buffer Addrs : Scope  NumInputPorts=8
      settings: TimeRange=2500  YMin=0~-1~0~0~0~0~-5~-5  YMax=1~1~1~150~70~1~5~5  SaveName=ScopeData54
  Inverter : xbsIndex_r4/Inverter  en=off  latency=0  dbl_ovrd=off  block_type=inv
      settings: block_version=VER_STRING_GOES_HERE
  Inverter1 : xbsIndex_r4/Inverter  en=off  latency=0  dbl_ovrd=off  block_type=inv
      settings: block_version=VER_STRING_GOES_HERE
  Inverter3 : xbsIndex_r4/Inverter  en=off  latency=0  dbl_ovrd=off  block_type=inv
      settings: block_version=VER_STRING_GOES_HERE
  Logical1 : xbsIndex_r4/Logical  logical_function=AND  inputs=2  en=off  latency=0  precision=Full  arith_type=Unsigned  n_bits=8  bin_pt=2  align_bp=on  dbl_ovrd=off  block_type=logical
      settings: xl_area=[0, 0, 0, 0, 0, 0, 0]  block_version=VER_STRING_GOES_HERE
  Logical2 : xbsIndex_r4/Logical  logical_function=OR  inputs=2  en=off  latency=0  precision=Full  arith_type=Unsigned  n_bits=8  bin_pt=2  align_bp=on  dbl_ovrd=off  block_type=logical
      settings: xl_area=[4 0 0 4 0 0 0]  block_version=VER_STRING_GOES_HERE
  Logical3 : xbsIndex_r4/Logical  logical_function=AND  inputs=3  en=off  latency=0  precision=Full  arith_type=Unsigned  n_bits=8  bin_pt=2  align_bp=on  dbl_ovrd=off  block_type=logical
      settings: xl_area=[0, 0, 0, 0, 0, 0, 0]  block_version=VER_STRING_GOES_HERE
  Logical4 : xbsIndex_r4/Logical  logical_function=AND  inputs=2  en=off  latency=0  precision=Full  arith_type=Unsigned  n_bits=8  bin_pt=2  align_bp=on  dbl_ovrd=off  block_type=logical
      settings: xl_area=[0, 0, 0, 0, 0, 0, 0]  block_version=VER_STRING_GOES_HERE
  Logical5 : xbsIndex_r4/Logical  logical_function=OR  inputs=2  en=off  latency=0  precision=Full  arith_type=Unsigned  n_bits=8  bin_pt=2  align_bp=on  dbl_ovrd=off  block_type=logical
      settings: xl_area=[0, 0, 0, 0, 0, 0, 0]  block_version=VER_STRING_GOES_HERE
  Logical6 : xbsIndex_r4/Logical  logical_function=AND  inputs=3  en=off  latency=0  precision=Full  arith_type=Unsigned  n_bits=8  bin_pt=2  align_bp=on  dbl_ovrd=off  block_type=logical
      settings: xl_area=[0, 0, 0, 0, 0, 0, 0]  block_version=VER_STRING_GOES_HERE
  Oscillator1 : Subsystem
  Posedge Det : Subsystem
  Relational : xbsIndex_r4/Relational  Orientation=left  mode=a<b  en=off  latency=0  dbl_ovrd=off  block_type=relational
      settings: block_version=VER_STRING_GOES_HERE
  S-R Latch : Subsystem
  done{1..4,7..10} (8 alike) : xbsIndex_r4/Gateway Out  hdl_port=off  timing_constraint=None  locs_specified=off  LOCs={}  block_type=gatewayout
      settings: infoedit="Gateway out block.  Converts Xilinx fixed point inputs into ouputs of type Simulink integer, double, or fixed point.<P><P>Hardware notes:  In hardware these blocks become top level output ports or are discarded, depending on how they are configured."  xl_area=[0 0 0 0 1 0 0]  block_version=VER_STRING_GOES_HERE
  Rd Addr : Outport
  Vout : Outport  Port=2
  wires:
  Counter/1 -> 6LSB/1, Relational/1, done10/1, 1MSB/1
  6LSB/1 -> Rd Addr/1, done2/1
  Constant1/1 -> Relational/2
  Relational/1 -> Logical1/2, Inverter/1
  Logical1/1 -> Counter/2, done7/1, Logical5/1, Delay2/1
  Posedge Det/1(Q) -> Logical2/1
  [rx_global_reset] -> Logical2/2
  Payload/1 -> Posedge Det/1(D), done1/1, Logical6/3
  done8/1 -> FFT Output Buffer Addrs/1
  done9/1 -> FFT Output Buffer Addrs/2
  done7/1 -> FFT Output Buffer Addrs/3
  done10/1 -> FFT Output Buffer Addrs/4
  done2/1 -> FFT Output Buffer Addrs/5
  Logical3/1 -> Counter/1
  Inverter/1 -> Logical3/3
  S-R Latch/1(D) -> Logical1/1
  Logical2/1 -> done9/1, S-R Latch/2(R), Oscillator1/2(rst)
  Logical4/1 -> Delay1/1
  FFT Done/1 -> done3/1, Oscillator1/1(en), Logical4/1
  NotTraining/1 -> done4/1, Logical4/2
  Delay2/1 -> Logical5/2
  Logical5/1 -> Logical6/2, Logical6/1
  done1/1 -> FFT Output Buffer Addrs/6
  1MSB/1 -> Inverter1/1
  Logical6/1 -> Vout/1
  done3/1 -> FFT Output Buffer Addrs/7
  done4/1 -> FFT Output Buffer Addrs/8
  Delay1/1 -> done8/1, S-R Latch/1(S), Logical3/2
  Oscillator1/1(Q) -> Inverter3/1
  Inverter3/1 -> Logical3/1

== system ofdm_txrx_alamouti/OFDM Rx Alamouti/FFT & Chan Est/Addr_Generator ==
  note: The memory read address is the FFT input index plus an offset in [0,max_cyclic_prefix]. This is how we strip
      | the cyclic prefix from the incoming sample stream- the FFT only gets numSubcarrier samples.
  note: The FFT core begins requesting input samples immediately
      | after its Start input is asserted. If Start is asserted again before
      | the first transform is complete, a second transform begins
      | immediately after the first. This is how we sequence the pairs
      | of transforms for the incoming OFDM symbols multiplexed between
      | antennas. The FFT is started as soon as the incoming sample stream
      | passes sample number (numSubcarriers/2)+symbolTimingOffset.
      | This is the lowest latency scheme, loading the FFT core with the
      | input OFDM symbol exactly 1 clock cycle after it's available.
  Payload : Inport
  FFT Xn_index : Inport  Port=2
  AddSub : xbsIndex_r4/AddSub  mode=Addition  use_carryin=off  use_carryout=off  en=off  latency=0  precision=User Defined  arith_type=Unsigned  n_bits=ceil(log2(max_num_subcarriers))+1  bin_pt=0  quantization=Truncate  overflow=Wrap  dbl_ovrd=off  use_behavioral_HDL=on  pipelined=off  use_rpm=on  block_type=addsub
      settings: xl_area=[4 0 0 6 0 0 0]  block_version=VER_STRING_GOES_HERE
  Assert : xbsIndex_r4/Assert  Orientation=left  assert_type=on  type_source=Explicitly  arith_type=Boolean  n_bits=16  bin_pt=14  assert_rate=off  rate_source=Explicitly  period=1  output_port=on  block_type=assert
      settings: infoedit=Asserts a user-defined sample rate and/or type on a signal.<P><P>Hardware notes: In hardware this block costs nothing.  block_version=VER_STRING_GOES_HERE
  Convert : xbsIndex_r4/Convert  arith_type=Unsigned  n_bits=ceil(log2(max_num_subcarriers))+1  bin_pt=0  quantization=Truncate  overflow=Wrap  latency=0  dbl_ovrd=off  pipeline=off  block_type=convert
      settings: block_version=VER_STRING_GOES_HERE
  Delay1 : xbsIndex_r4/Delay  en=off  latency=1  dbl_ovrd=off  reg_retiming=off  block_type=delay
      settings: infoedit=Hardware notes: A delay line is a chain, each link of which is an SRL16 followed by a flip-flop. If register retiming is enabled, the delay line is a chain of flip-flops.  block_version=VER_STRING_GOES_HERE
  Down Sample : xbsIndex_r4/Down Sample  Orientation=left  sample_ratio=2  sample_phase="Last Value of Frame  (most efficient)"  en=off  latency=1  dbl_ovrd=off  block_type=dsamp
      settings: block_version=VER_STRING_GOES_HERE
  FFT Start Gen : Subsystem
  Inverter : xbsIndex_r4/Inverter  Orientation=left  en=off  latency=0  dbl_ovrd=off  block_type=inv
      settings: block_version=VER_STRING_GOES_HERE
  Logical1 : xbsIndex_r4/Logical  logical_function=OR  inputs=2  en=off  latency=0  precision=Full  arith_type=Unsigned  n_bits=16  bin_pt=0  align_bp=on  dbl_ovrd=off  block_type=logical
      settings: block_version=VER_STRING_GOES_HERE
  Logical2 : xbsIndex_r4/Logical  logical_function=AND  inputs=2  en=off  latency=0  precision=Full  arith_type=Unsigned  n_bits=16  bin_pt=0  align_bp=on  dbl_ovrd=off  block_type=logical
      settings: block_version=VER_STRING_GOES_HERE
  Logical3 : xbsIndex_r4/Logical  logical_function=OR  inputs=2  en=off  latency=0  precision=Full  arith_type=Unsigned  n_bits=16  bin_pt=0  align_bp=on  dbl_ovrd=off  block_type=logical
      settings: block_version=VER_STRING_GOES_HERE
  MSB : xbsIndex_r4/Slice  nbits=1  boolean_output=on  mode=Upper Bit Location + Width  bit1=0  base1=MSB of Input  bit0=0  base0=LSB of Input  dbl_ovrd=off  block_type=slice
      settings: infoedit="Extracts a given range of bits from each input sample and presents it at the output.  The output type is ordinarily unsigned with binary point at zero, but can be Boolean when the slice is one bit wide.<P><P>Hardware notes: In hardware this block costs nothing."  block_version=VER_STRING_GOES_HERE
  Oscillator : Subsystem
  Posedge : Subsystem
  Register : xbsIndex_r4/Register  init=0  rst=on  en=on  dbl_ovrd=off  block_type=register
      settings: block_version=VER_STRING_GOES_HERE
  Relational : xbsIndex_r4/Relational  Orientation=left  mode=a=b  en=off  latency=0  dbl_ovrd=off  block_type=relational
      settings: block_version=VER_STRING_GOES_HERE
  Rx Addr Gen : Scope  NumInputPorts=5
      settings: TimeRange=4000  YMin=0~0~0~0~0  YMax=1~80~1~80~80  SaveName=ScopeData4
  Samp Counter : xbsIndex_r4/Counter  cnt_type=Free Running  cnt_to=79  operation=Up  start_count=0  cnt_by_val=1  arith_type=Unsigned  n_bits=ceil(log2(max_num_subcarriers + max_CP_length))  bin_pt=0  load_pin=off  rst=on  en=on  explicit_period=off  period=1  dbl_ovrd=off  use_behavioral_HDL=off  use_rpm=on  block_type=counter
      settings: xl_area=[5 7 0 9 0 0 0]  block_version=VER_STRING_GOES_HERE
  done{1,3..6} (5 alike) : xbsIndex_r4/Gateway Out  hdl_port=off  timing_constraint=None  locs_specified=off  LOCs={}  block_type=gatewayout
      settings: infoedit="Gateway out block.  Converts Xilinx fixed point inputs into ouputs of type Simulink integer, double, or fixed point.<P><P>Hardware notes:  In hardware these blocks become top level output ports or are discarded, depending on how they are configured."  xl_area=[0 0 0 0 1 0 0]  block_version=VER_STRING_GOES_HERE
  negedge : xbsIndex_r4/Expression  expression=~a & b  align_bp=on  en=off  latency=0  precision=Full  arith_type=Unsigned  n_bits=16  bin_pt=0  dbl_ovrd=off  block_type=expr
      settings: block_version=VER_STRING_GOES_HERE
  Write Addr : Outport
  Read Addr : Outport  Port=2
  Ant Sel : Outport  Port=3
  Wr Slot : Outport  Port=4
  FFT Start : Outport  Port=5
  wires:
  Convert/1 -> AddSub/1
  [regRx_symbolTimingOffset] -> AddSub/2
  [regRX_sampsPerSymMinusOne] -> Down Sample/1
  Samp Counter/1 -> Write Addr/1, FFT Start Gen/1(WriteAddr), Relational/2, done5/1
  Relational/1 -> Logical3/1, Oscillator/1(en)
  Posedge/1(Q) -> Logical1/1
  Logical1/1 -> [localReset]
  Payload/1 -> Posedge/1(D), Samp Counter/2, done4/1, Logical2/1
  Logical3/1 -> Samp Counter/1
  [localReset] -> Logical3/2, Oscillator/2(rst)
  [rx_global_reset_DV2] -> Logical1/2
  AddSub/1 -> Read Addr/1, done6/1
  done4/1 -> Rx Addr Gen/1
  done5/1 -> Rx Addr Gen/2
  done3/1 -> Rx Addr Gen/3
  done6/1 -> Rx Addr Gen/4
  done1/1 -> Rx Addr Gen/5
  Down Sample/1 -> Relational/1
  FFT Xn_index/1 -> Convert/1, MSB/1
  FFT Start Gen/1(Start) -> done3/1, Logical2/2
  Delay1/1 -> negedge/2
  MSB/1 -> negedge/1, Delay1/1
  Register/1 -> Assert/1, Ant Sel/1, done1/1
  Inverter/1 -> Register/1
  Assert/1 -> Inverter/1
  Oscillator/1(Q) -> Wr Slot/1, FFT Start Gen/2(Wr Slot)
  negedge/1 -> Register/3
  [localReset] -> Register/2
  Logical2/1 -> FFT Start/1
